simulink model "SOC_2023b"  (R2023b)
settings: Solver=VariableStepAuto  StartTime=0.0  StopTime=10.0  RelTol=1e-3
undefined symbols (not defined in the file or in its folder): Initial_SOC
(part 3 of 187)

== system SOC_2023b/SOC/Multi-Temperature SOC EKF1 ==
  note: McMaster Automotive Research Centre - MARC
      | Electrical Engineer: Romulo Navega Vieira, M.Sc and MBA - PhD Candidate
  Current : Inport
  Voltage : Inport  Port=2
  Temperature : Inport  Port=3
  Data Store Write1 : DataStoreWrite  DataStoreName=HVBat_SOCcell001  IndexOptions=Index vector (dialog)  Indices=1  OutputSizes=1
  Extended Kalman Filter - Multi Temperature EKF : Subsystem
  Gain : Gain  Gain=100
  Saturation : Saturate  UpperLimit=100  LowerLimit=0
  HVBat_SOCcell001 : Outport
  wires:
  Extended Kalman Filter - Multi Temperature EKF/1(SOC) -> Gain/1
  Voltage/1 -> Extended Kalman Filter - Multi Temperature EKF/2(Voltage)
  Current/1 -> Extended Kalman Filter - Multi Temperature EKF/1(Current)
  Temperature/1 -> Extended Kalman Filter - Multi Temperature EKF/3(Temperature)
  Gain/1 -> Saturation/1
  Saturation/1 -> HVBat_SOCcell001/1, Data Store Write1/1

== system SOC_2023b/SOC/Multi-Temperature SOC EKF10 ==
  note: McMaster Automotive Research Centre - MARC
      | Electrical Engineer: Romulo Navega Vieira, M.Sc and MBA - PhD Candidate
  Current : Inport
  Voltage : Inport  Port=2
  Temperature : Inport  Port=3
  Data Store Write1 : DataStoreWrite  DataStoreName=HVBat_SOCcell010  IndexOptions=Index vector (dialog)  Indices=1  OutputSizes=1
  Extended Kalman Filter - Multi Temperature EKF : Subsystem
  Gain : Gain  Gain=100
  Saturation : Saturate  UpperLimit=100  LowerLimit=0
  HVBat_SOCcell010 : Outport
  wires:
  Voltage/1 -> Extended Kalman Filter - Multi Temperature EKF/2(Voltage)
  Current/1 -> Extended Kalman Filter - Multi Temperature EKF/1(Current)
  Temperature/1 -> Extended Kalman Filter - Multi Temperature EKF/3(Temperature)
  Extended Kalman Filter - Multi Temperature EKF/1(SOC) -> Gain/1
  Gain/1 -> Saturation/1
  Saturation/1 -> HVBat_SOCcell010/1, Data Store Write1/1

== system SOC_2023b/SOC/Multi-Temperature SOC EKF100 ==
  note: McMaster Automotive Research Centre - MARC
      | Electrical Engineer: Romulo Navega Vieira, M.Sc and MBA - PhD Candidate
  Current : Inport
  Voltage : Inport  Port=2
  Temperature : Inport  Port=3
  Data Store Write1 : DataStoreWrite  DataStoreName=HVBat_SOCcell100  IndexOptions=Index vector (dialog)  Indices=1  OutputSizes=1
  Extended Kalman Filter - Multi Temperature EKF : Subsystem
  Gain : Gain  Gain=100
  Saturation : Saturate  UpperLimit=100  LowerLimit=0
  HVBat_SOCcell100 : Outport
  wires:
  Voltage/1 -> Extended Kalman Filter - Multi Temperature EKF/2(Voltage)
  Current/1 -> Extended Kalman Filter - Multi Temperature EKF/1(Current)
  Temperature/1 -> Extended Kalman Filter - Multi Temperature EKF/3(Temperature)
  Extended Kalman Filter - Multi Temperature EKF/1(SOC) -> Gain/1
  Gain/1 -> Saturation/1
  Saturation/1 -> HVBat_SOCcell100/1, Data Store Write1/1

== system SOC_2023b/SOC/Multi-Temperature SOC EKF101 ==
  note: McMaster Automotive Research Centre - MARC
      | Electrical Engineer: Romulo Navega Vieira, M.Sc and MBA - PhD Candidate
  Current : Inport
  Voltage : Inport  Port=2
  Temperature : Inport  Port=3
  Data Store Write1 : DataStoreWrite  DataStoreName=HVBat_SOCcell101  IndexOptions=Index vector (dialog)  Indices=1  OutputSizes=1
  Extended Kalman Filter - Multi Temperature EKF : Subsystem
  Gain : Gain  Gain=100
  Saturation : Saturate  UpperLimit=100  LowerLimit=0
  HVBat_SOCcell101 : Outport
  wires:
  Voltage/1 -> Extended Kalman Filter - Multi Temperature EKF/2(Voltage)
  Current/1 -> Extended Kalman Filter - Multi Temperature EKF/1(Current)
  Temperature/1 -> Extended Kalman Filter - Multi Temperature EKF/3(Temperature)
  Extended Kalman Filter - Multi Temperature EKF/1(SOC) -> Gain/1
  Gain/1 -> Saturation/1
  Saturation/1 -> HVBat_SOCcell101/1, Data Store Write1/1

== system SOC_2023b/SOC/Multi-Temperature SOC EKF102 ==
  note: McMaster Automotive Research Centre - MARC
      | Electrical Engineer: Romulo Navega Vieira, M.Sc and MBA - PhD Candidate
  Current : Inport
  Voltage : Inport  Port=2
  Temperature : Inport  Port=3
  Data Store Write1 : DataStoreWrite  DataStoreName=HVBat_SOCcell102  IndexOptions=Index vector (dialog)  Indices=1  OutputSizes=1
  Extended Kalman Filter - Multi Temperature EKF : Subsystem
  Gain : Gain  Gain=100
  Saturation : Saturate  UpperLimit=100  LowerLimit=0
  HVBat_SOCcell102 : Outport
  wires:
  Voltage/1 -> Extended Kalman Filter - Multi Temperature EKF/2(Voltage)
  Current/1 -> Extended Kalman Filter - Multi Temperature EKF/1(Current)
  Temperature/1 -> Extended Kalman Filter - Multi Temperature EKF/3(Temperature)
  Extended Kalman Filter - Multi Temperature EKF/1(SOC) -> Gain/1
  Gain/1 -> Saturation/1
  Saturation/1 -> HVBat_SOCcell102/1, Data Store Write1/1

== system SOC_2023b/SOC/Multi-Temperature SOC EKF103 ==
  note: McMaster Automotive Research Centre - MARC
      | Electrical Engineer: Romulo Navega Vieira, M.Sc and MBA - PhD Candidate
  Current : Inport
  Voltage : Inport  Port=2
  Temperature : Inport  Port=3
  Data Store Write1 : DataStoreWrite  DataStoreName=HVBat_SOCcell103  IndexOptions=Index vector (dialog)  Indices=1  OutputSizes=1
  Extended Kalman Filter - Multi Temperature EKF : Subsystem
  Gain : Gain  Gain=100
  Saturation : Saturate  UpperLimit=100  LowerLimit=0
  HVBat_SOCcell103 : Outport
  wires:
  Voltage/1 -> Extended Kalman Filter - Multi Temperature EKF/2(Voltage)
  Current/1 -> Extended Kalman Filter - Multi Temperature EKF/1(Current)
  Temperature/1 -> Extended Kalman Filter - Multi Temperature EKF/3(Temperature)
  Extended Kalman Filter - Multi Temperature EKF/1(SOC) -> Gain/1
  Gain/1 -> Saturation/1
  Saturation/1 -> HVBat_SOCcell103/1, Data Store Write1/1

== system SOC_2023b/SOC/Multi-Temperature SOC EKF104 ==
  note: McMaster Automotive Research Centre - MARC
      | Electrical Engineer: Romulo Navega Vieira, M.Sc and MBA - PhD Candidate
  Current : Inport
  Voltage : Inport  Port=2
  Temperature : Inport  Port=3
  Data Store Write1 : DataStoreWrite  DataStoreName=HVBat_SOCcell104  IndexOptions=Index vector (dialog)  Indices=1  OutputSizes=1
  Extended Kalman Filter - Multi Temperature EKF : Subsystem
  Gain : Gain  Gain=100
  Saturation : Saturate  UpperLimit=100  LowerLimit=0
  HVBat_SOCcell104 : Outport
  wires:
  Voltage/1 -> Extended Kalman Filter - Multi Temperature EKF/2(Voltage)
  Current/1 -> Extended Kalman Filter - Multi Temperature EKF/1(Current)
  Temperature/1 -> Extended Kalman Filter - Multi Temperature EKF/3(Temperature)
  Extended Kalman Filter - Multi Temperature EKF/1(SOC) -> Gain/1
  Gain/1 -> Saturation/1
  Saturation/1 -> HVBat_SOCcell104/1, Data Store Write1/1

== system SOC_2023b/SOC/Multi-Temperature SOC EKF105 ==
  note: McMaster Automotive Research Centre - MARC
      | Electrical Engineer: Romulo Navega Vieira, M.Sc and MBA - PhD Candidate
  Current : Inport
  Voltage : Inport  Port=2
  Temperature : Inport  Port=3
  Data Store Write1 : DataStoreWrite  DataStoreName=HVBat_SOCcell105  IndexOptions=Index vector (dialog)  Indices=1  OutputSizes=1
  Extended Kalman Filter - Multi Temperature EKF : Subsystem
  Gain : Gain  Gain=100
  Saturation : Saturate  UpperLimit=100  LowerLimit=0
  HVBat_SOCcell105 : Outport
  wires:
  Voltage/1 -> Extended Kalman Filter - Multi Temperature EKF/2(Voltage)
  Current/1 -> Extended Kalman Filter - Multi Temperature EKF/1(Current)
  Temperature/1 -> Extended Kalman Filter - Multi Temperature EKF/3(Temperature)
  Extended Kalman Filter - Multi Temperature EKF/1(SOC) -> Gain/1
  Gain/1 -> Saturation/1
  Saturation/1 -> HVBat_SOCcell105/1, Data Store Write1/1

== system SOC_2023b/SOC/Multi-Temperature SOC EKF11 ==
  note: McMaster Automotive Research Centre - MARC
      | Electrical Engineer: Romulo Navega Vieira, M.Sc and MBA - PhD Candidate
  Current : Inport
  Voltage : Inport  Port=2
  Temperature : Inport  Port=3
  Data Store Write1 : DataStoreWrite  DataStoreName=HVBat_SOCcell011  IndexOptions=Index vector (dialog)  Indices=1  OutputSizes=1
  Extended Kalman Filter - Multi Temperature EKF : Subsystem
  Gain : Gain  Gain=100
  Saturation : Saturate  UpperLimit=100  LowerLimit=0
  HVBat_SOCcell011 : Outport
  wires:
  Voltage/1 -> Extended Kalman Filter - Multi Temperature EKF/2(Voltage)
  Current/1 -> Extended Kalman Filter - Multi Temperature EKF/1(Current)
  Temperature/1 -> Extended Kalman Filter - Multi Temperature EKF/3(Temperature)
  Extended Kalman Filter - Multi Temperature EKF/1(SOC) -> Gain/1
  Gain/1 -> Saturation/1
  Saturation/1 -> HVBat_SOCcell011/1, Data Store Write1/1

== system SOC_2023b/SOC/Multi-Temperature SOC EKF12 ==
  note: McMaster Automotive Research Centre - MARC
      | Electrical Engineer: Romulo Navega Vieira, M.Sc and MBA - PhD Candidate
  Current : Inport
  Voltage : Inport  Port=2
  Temperature : Inport  Port=3
  Data Store Write1 : DataStoreWrite  DataStoreName=HVBat_SOCcell012  IndexOptions=Index vector (dialog)  Indices=1  OutputSizes=1
  Extended Kalman Filter - Multi Temperature EKF : Subsystem
  Gain : Gain  Gain=100
  Saturation : Saturate  UpperLimit=100  LowerLimit=0
  HVBat_SOCcell012 : Outport
  wires:
  Voltage/1 -> Extended Kalman Filter - Multi Temperature EKF/2(Voltage)
  Current/1 -> Extended Kalman Filter - Multi Temperature EKF/1(Current)
  Temperature/1 -> Extended Kalman Filter - Multi Temperature EKF/3(Temperature)
  Extended Kalman Filter - Multi Temperature EKF/1(SOC) -> Gain/1
  Gain/1 -> Saturation/1
  Saturation/1 -> HVBat_SOCcell012/1, Data Store Write1/1

== system SOC_2023b/SOC/Multi-Temperature SOC EKF13 ==
  note: McMaster Automotive Research Centre - MARC
      | Electrical Engineer: Romulo Navega Vieira, M.Sc and MBA - PhD Candidate
  Current : Inport
  Voltage : Inport  Port=2
  Temperature : Inport  Port=3
  Data Store Write1 : DataStoreWrite  DataStoreName=HVBat_SOCcell013  IndexOptions=Index vector (dialog)  Indices=1  OutputSizes=1
  Extended Kalman Filter - Multi Temperature EKF : Subsystem
  Gain : Gain  Gain=100
  Saturation : Saturate  UpperLimit=100  LowerLimit=0
  HVBat_SOCcell013 : Outport
  wires:
  Voltage/1 -> Extended Kalman Filter - Multi Temperature EKF/2(Voltage)
  Current/1 -> Extended Kalman Filter - Multi Temperature EKF/1(Current)
  Temperature/1 -> Extended Kalman Filter - Multi Temperature EKF/3(Temperature)
  Extended Kalman Filter - Multi Temperature EKF/1(SOC) -> Gain/1
  Gain/1 -> Saturation/1
  Saturation/1 -> HVBat_SOCcell013/1, Data Store Write1/1

== system SOC_2023b/SOC/Multi-Temperature SOC EKF14 ==
  note: McMaster Automotive Research Centre - MARC
      | Electrical Engineer: Romulo Navega Vieira, M.Sc and MBA - PhD Candidate
  Current : Inport
  Voltage : Inport  Port=2
  Temperature : Inport  Port=3
  Data Store Write1 : DataStoreWrite  DataStoreName=HVBat_SOCcell014  IndexOptions=Index vector (dialog)  Indices=1  OutputSizes=1
  Extended Kalman Filter - Multi Temperature EKF : Subsystem
  Gain : Gain  Gain=100
  Saturation : Saturate  UpperLimit=100  LowerLimit=0
  HVBat_SOCcell014 : Outport
  wires:
  Voltage/1 -> Extended Kalman Filter - Multi Temperature EKF/2(Voltage)
  Current/1 -> Extended Kalman Filter - Multi Temperature EKF/1(Current)
  Temperature/1 -> Extended Kalman Filter - Multi Temperature EKF/3(Temperature)
  Extended Kalman Filter - Multi Temperature EKF/1(SOC) -> Gain/1
  Gain/1 -> Saturation/1
  Saturation/1 -> HVBat_SOCcell014/1, Data Store Write1/1

== system SOC_2023b/SOC/Multi-Temperature SOC EKF15 ==
  note: McMaster Automotive Research Centre - MARC
      | Electrical Engineer: Romulo Navega Vieira, M.Sc and MBA - PhD Candidate
  Current : Inport
  Voltage : Inport  Port=2
  Temperature : Inport  Port=3
  Data Store Write1 : DataStoreWrite  DataStoreName=HVBat_SOCcell015  IndexOptions=Index vector (dialog)  Indices=1  OutputSizes=1
  Extended Kalman Filter - Multi Temperature EKF : Subsystem
  Gain : Gain  Gain=100
  Saturation : Saturate  UpperLimit=100  LowerLimit=0
  HVBat_SOCcell015 : Outport
  wires:
  Voltage/1 -> Extended Kalman Filter - Multi Temperature EKF/2(Voltage)
  Current/1 -> Extended Kalman Filter - Multi Temperature EKF/1(Current)
  Temperature/1 -> Extended Kalman Filter - Multi Temperature EKF/3(Temperature)
  Extended Kalman Filter - Multi Temperature EKF/1(SOC) -> Gain/1
  Gain/1 -> Saturation/1
  Saturation/1 -> HVBat_SOCcell015/1, Data Store Write1/1

== system SOC_2023b/SOC/Multi-Temperature SOC EKF16 ==
  note: McMaster Automotive Research Centre - MARC
      | Electrical Engineer: Romulo Navega Vieira, M.Sc and MBA - PhD Candidate
  Current : Inport
  Voltage : Inport  Port=2
  Temperature : Inport  Port=3
  Data Store Write1 : DataStoreWrite  DataStoreName=HVBat_SOCcell016  IndexOptions=Index vector (dialog)  Indices=1  OutputSizes=1
  Extended Kalman Filter - Multi Temperature EKF : Subsystem
  Gain : Gain  Gain=100
  Saturation : Saturate  UpperLimit=100  LowerLimit=0
  HVBat_SOCcell016 : Outport
  wires:
  Voltage/1 -> Extended Kalman Filter - Multi Temperature EKF/2(Voltage)
  Current/1 -> Extended Kalman Filter - Multi Temperature EKF/1(Current)
  Temperature/1 -> Extended Kalman Filter - Multi Temperature EKF/3(Temperature)
  Extended Kalman Filter - Multi Temperature EKF/1(SOC) -> Gain/1
  Gain/1 -> Saturation/1
  Saturation/1 -> HVBat_SOCcell016/1, Data Store Write1/1

== system SOC_2023b/SOC/Multi-Temperature SOC EKF17 ==
  note: McMaster Automotive Research Centre - MARC
      | Electrical Engineer: Romulo Navega Vieira, M.Sc and MBA - PhD Candidate
  Current : Inport
  Voltage : Inport  Port=2
  Temperature : Inport  Port=3
  Data Store Write1 : DataStoreWrite  DataStoreName=HVBat_SOCcell017  IndexOptions=Index vector (dialog)  Indices=1  OutputSizes=1
  Extended Kalman Filter - Multi Temperature EKF : Subsystem
  Gain : Gain  Gain=100
  Saturation : Saturate  UpperLimit=100  LowerLimit=0
  HVBat_SOCcell017 : Outport
  wires:
  Voltage/1 -> Extended Kalman Filter - Multi Temperature EKF/2(Voltage)
  Current/1 -> Extended Kalman Filter - Multi Temperature EKF/1(Current)
  Temperature/1 -> Extended Kalman Filter - Multi Temperature EKF/3(Temperature)
  Extended Kalman Filter - Multi Temperature EKF/1(SOC) -> Gain/1
  Gain/1 -> Saturation/1
  Saturation/1 -> HVBat_SOCcell017/1, Data Store Write1/1

== system SOC_2023b/SOC/Multi-Temperature SOC EKF18 ==
  note: McMaster Automotive Research Centre - MARC
      | Electrical Engineer: Romulo Navega Vieira, M.Sc and MBA - PhD Candidate
  Current : Inport
  Voltage : Inport  Port=2
  Temperature : Inport  Port=3
  Data Store Write1 : DataStoreWrite  DataStoreName=HVBat_SOCcell018  IndexOptions=Index vector (dialog)  Indices=1  OutputSizes=1
  Extended Kalman Filter - Multi Temperature EKF : Subsystem
  Gain : Gain  Gain=100
  Saturation : Saturate  UpperLimit=100  LowerLimit=0
  HVBat_SOCcell018 : Outport
  wires:
  Voltage/1 -> Extended Kalman Filter - Multi Temperature EKF/2(Voltage)
  Current/1 -> Extended Kalman Filter - Multi Temperature EKF/1(Current)
  Temperature/1 -> Extended Kalman Filter - Multi Temperature EKF/3(Temperature)
  Extended Kalman Filter - Multi Temperature EKF/1(SOC) -> Gain/1
  Gain/1 -> Saturation/1
  Saturation/1 -> HVBat_SOCcell018/1, Data Store Write1/1

== system SOC_2023b/SOC/Multi-Temperature SOC EKF19 ==
  note: McMaster Automotive Research Centre - MARC
      | Electrical Engineer: Romulo Navega Vieira, M.Sc and MBA - PhD Candidate
  Current : Inport
  Voltage : Inport  Port=2
  Temperature : Inport  Port=3
  Data Store Write1 : DataStoreWrite  DataStoreName=HVBat_SOCcell019  IndexOptions=Index vector (dialog)  Indices=1  OutputSizes=1
  Extended Kalman Filter - Multi Temperature EKF : Subsystem
  Gain : Gain  Gain=100
  Saturation : Saturate  UpperLimit=100  LowerLimit=0
  HVBat_SOCcell019 : Outport
  wires:
  Voltage/1 -> Extended Kalman Filter - Multi Temperature EKF/2(Voltage)
  Current/1 -> Extended Kalman Filter - Multi Temperature EKF/1(Current)
  Temperature/1 -> Extended Kalman Filter - Multi Temperature EKF/3(Temperature)
  Extended Kalman Filter - Multi Temperature EKF/1(SOC) -> Gain/1
  Gain/1 -> Saturation/1
  Saturation/1 -> HVBat_SOCcell019/1, Data Store Write1/1

== system SOC_2023b/SOC/Multi-Temperature SOC EKF2 ==
  note: McMaster Automotive Research Centre - MARC
      | Electrical Engineer: Romulo Navega Vieira, M.Sc and MBA - PhD Candidate
  Current : Inport
  Voltage : Inport  Port=2
  Temperature : Inport  Port=3
  Data Store Write1 : DataStoreWrite  DataStoreName=HVBat_SOCcell002  IndexOptions=Index vector (dialog)  Indices=1  OutputSizes=1
  Extended Kalman Filter - Multi Temperature EKF : Subsystem
  Gain : Gain  Gain=100
  Saturation : Saturate  UpperLimit=100  LowerLimit=0
  HVBat_SOCcell002 : Outport
  wires:
  Voltage/1 -> Extended Kalman Filter - Multi Temperature EKF/2(Voltage)
  Current/1 -> Extended Kalman Filter - Multi Temperature EKF/1(Current)
  Temperature/1 -> Extended Kalman Filter - Multi Temperature EKF/3(Temperature)
  Extended Kalman Filter - Multi Temperature EKF/1(SOC) -> Gain/1
  Gain/1 -> Saturation/1
  Saturation/1 -> HVBat_SOCcell002/1, Data Store Write1/1

== system SOC_2023b/SOC/Multi-Temperature SOC EKF20 ==
  note: McMaster Automotive Research Centre - MARC
      | Electrical Engineer: Romulo Navega Vieira, M.Sc and MBA - PhD Candidate
  Current : Inport
  Voltage : Inport  Port=2
  Temperature : Inport  Port=3
  Data Store Write1 : DataStoreWrite  DataStoreName=HVBat_SOCcell020  IndexOptions=Index vector (dialog)  Indices=1  OutputSizes=1
  Extended Kalman Filter - Multi Temperature EKF : Subsystem
  Gain : Gain  Gain=100
  Saturation : Saturate  UpperLimit=100  LowerLimit=0
  HVBat_SOCcell020 : Outport
  wires:
  Voltage/1 -> Extended Kalman Filter - Multi Temperature EKF/2(Voltage)
  Current/1 -> Extended Kalman Filter - Multi Temperature EKF/1(Current)
  Temperature/1 -> Extended Kalman Filter - Multi Temperature EKF/3(Temperature)
  Extended Kalman Filter - Multi Temperature EKF/1(SOC) -> Gain/1
  Gain/1 -> Saturation/1
  Saturation/1 -> HVBat_SOCcell020/1, Data Store Write1/1

== system SOC_2023b/SOC/Multi-Temperature SOC EKF21 ==
  note: McMaster Automotive Research Centre - MARC
      | Electrical Engineer: Romulo Navega Vieira, M.Sc and MBA - PhD Candidate
  Current : Inport
  Voltage : Inport  Port=2
  Temperature : Inport  Port=3
  Data Store Write1 : DataStoreWrite  DataStoreName=HVBat_SOCcell021  IndexOptions=Index vector (dialog)  Indices=1  OutputSizes=1
  Extended Kalman Filter - Multi Temperature EKF : Subsystem
  Gain : Gain  Gain=100
  Saturation : Saturate  UpperLimit=100  LowerLimit=0
  HVBat_SOCcell021 : Outport
  wires:
  Extended Kalman Filter - Multi Temperature EKF/1(SOC) -> Gain/1
  Voltage/1 -> Extended Kalman Filter - Multi Temperature EKF/2(Voltage)
  Current/1 -> Extended Kalman Filter - Multi Temperature EKF/1(Current)
  Temperature/1 -> Extended Kalman Filter - Multi Temperature EKF/3(Temperature)
  Gain/1 -> Saturation/1
  Saturation/1 -> HVBat_SOCcell021/1, Data Store Write1/1

== system SOC_2023b/SOC/Multi-Temperature SOC EKF22 ==
  note: McMaster Automotive Research Centre - MARC
      | Electrical Engineer: Romulo Navega Vieira, M.Sc and MBA - PhD Candidate
  Current : Inport
  Voltage : Inport  Port=2
  Temperature : Inport  Port=3
  Data Store Write1 : DataStoreWrite  DataStoreName=HVBat_SOCcell022  IndexOptions=Index vector (dialog)  Indices=1  OutputSizes=1
  Extended Kalman Filter - Multi Temperature EKF : Subsystem
  Gain : Gain  Gain=100
  Saturation : Saturate  UpperLimit=100  LowerLimit=0
  HVBat_SOCcell022 : Outport
  wires:
  Voltage/1 -> Extended Kalman Filter - Multi Temperature EKF/2(Voltage)
  Current/1 -> Extended Kalman Filter - Multi Temperature EKF/1(Current)
  Temperature/1 -> Extended Kalman Filter - Multi Temperature EKF/3(Temperature)
  Extended Kalman Filter - Multi Temperature EKF/1(SOC) -> Gain/1
  Gain/1 -> Saturation/1
  Saturation/1 -> HVBat_SOCcell022/1, Data Store Write1/1

== system SOC_2023b/SOC/Multi-Temperature SOC EKF23 ==
  note: McMaster Automotive Research Centre - MARC
      | Electrical Engineer: Romulo Navega Vieira, M.Sc and MBA - PhD Candidate
  Current : Inport
  Voltage : Inport  Port=2
  Temperature : Inport  Port=3
  Data Store Write1 : DataStoreWrite  DataStoreName=HVBat_SOCcell023  IndexOptions=Index vector (dialog)  Indices=1  OutputSizes=1
  Extended Kalman Filter - Multi Temperature EKF : Subsystem
  Gain : Gain  Gain=100
  Saturation : Saturate  UpperLimit=100  LowerLimit=0
  HVBat_SOCcell023 : Outport
  wires:
  Voltage/1 -> Extended Kalman Filter - Multi Temperature EKF/2(Voltage)
  Current/1 -> Extended Kalman Filter - Multi Temperature EKF/1(Current)
  Temperature/1 -> Extended Kalman Filter - Multi Temperature EKF/3(Temperature)
  Extended Kalman Filter - Multi Temperature EKF/1(SOC) -> Gain/1
  Gain/1 -> Saturation/1
  Saturation/1 -> HVBat_SOCcell023/1, Data Store Write1/1

== system SOC_2023b/SOC/Multi-Temperature SOC EKF24 ==
  note: McMaster Automotive Research Centre - MARC
      | Electrical Engineer: Romulo Navega Vieira, M.Sc and MBA - PhD Candidate
  Current : Inport
  Voltage : Inport  Port=2
  Temperature : Inport  Port=3
  Data Store Write1 : DataStoreWrite  DataStoreName=HVBat_SOCcell024  IndexOptions=Index vector (dialog)  Indices=1  OutputSizes=1
  Extended Kalman Filter - Multi Temperature EKF : Subsystem
  Gain : Gain  Gain=100
  Saturation : Saturate  UpperLimit=100  LowerLimit=0
  HVBat_SOCcell024 : Outport
  wires:
  Voltage/1 -> Extended Kalman Filter - Multi Temperature EKF/2(Voltage)
  Current/1 -> Extended Kalman Filter - Multi Temperature EKF/1(Current)
  Temperature/1 -> Extended Kalman Filter - Multi Temperature EKF/3(Temperature)
  Extended Kalman Filter - Multi Temperature EKF/1(SOC) -> Gain/1
  Gain/1 -> Saturation/1
  Saturation/1 -> HVBat_SOCcell024/1, Data Store Write1/1

== system SOC_2023b/SOC/Multi-Temperature SOC EKF25 ==
  note: McMaster Automotive Research Centre - MARC
      | Electrical Engineer: Romulo Navega Vieira, M.Sc and MBA - PhD Candidate
  Current : Inport
  Voltage : Inport  Port=2
  Temperature : Inport  Port=3
  Data Store Write1 : DataStoreWrite  DataStoreName=HVBat_SOCcell025  IndexOptions=Index vector (dialog)  Indices=1  OutputSizes=1
  Extended Kalman Filter - Multi Temperature EKF : Subsystem
  Gain : Gain  Gain=100
  Saturation : Saturate  UpperLimit=100  LowerLimit=0
  HVBat_SOCcell025 : Outport
  wires:
  Voltage/1 -> Extended Kalman Filter - Multi Temperature EKF/2(Voltage)
  Current/1 -> Extended Kalman Filter - Multi Temperature EKF/1(Current)
  Temperature/1 -> Extended Kalman Filter - Multi Temperature EKF/3(Temperature)
  Extended Kalman Filter - Multi Temperature EKF/1(SOC) -> Gain/1
  Gain/1 -> Saturation/1
  Saturation/1 -> HVBat_SOCcell025/1, Data Store Write1/1

== system SOC_2023b/SOC/Multi-Temperature SOC EKF26 ==
  note: McMaster Automotive Research Centre - MARC
      | Electrical Engineer: Romulo Navega Vieira, M.Sc and MBA - PhD Candidate
  Current : Inport
  Voltage : Inport  Port=2
  Temperature : Inport  Port=3
  Data Store Write1 : DataStoreWrite  DataStoreName=HVBat_SOCcell026  IndexOptions=Index vector (dialog)  Indices=1  OutputSizes=1
  Extended Kalman Filter - Multi Temperature EKF : Subsystem
  Gain : Gain  Gain=100
  Saturation : Saturate  UpperLimit=100  LowerLimit=0
  HVBat_SOCcell026 : Outport
  wires:
  Voltage/1 -> Extended Kalman Filter - Multi Temperature EKF/2(Voltage)
  Current/1 -> Extended Kalman Filter - Multi Temperature EKF/1(Current)
  Temperature/1 -> Extended Kalman Filter - Multi Temperature EKF/3(Temperature)
  Extended Kalman Filter - Multi Temperature EKF/1(SOC) -> Gain/1
  Gain/1 -> Saturation/1
  Saturation/1 -> HVBat_SOCcell026/1, Data Store Write1/1

== system SOC_2023b/SOC/Multi-Temperature SOC EKF27 ==
  note: McMaster Automotive Research Centre - MARC
      | Electrical Engineer: Romulo Navega Vieira, M.Sc and MBA - PhD Candidate
  Current : Inport
  Voltage : Inport  Port=2
  Temperature : Inport  Port=3
  Data Store Write1 : DataStoreWrite  DataStoreName=HVBat_SOCcell027  IndexOptions=Index vector (dialog)  Indices=1  OutputSizes=1
  Extended Kalman Filter - Multi Temperature EKF : Subsystem
  Gain : Gain  Gain=100
  Saturation : Saturate  UpperLimit=100  LowerLimit=0
  HVBat_SOCcell027 : Outport
  wires:
  Voltage/1 -> Extended Kalman Filter - Multi Temperature EKF/2(Voltage)
  Current/1 -> Extended Kalman Filter - Multi Temperature EKF/1(Current)
  Temperature/1 -> Extended Kalman Filter - Multi Temperature EKF/3(Temperature)
  Extended Kalman Filter - Multi Temperature EKF/1(SOC) -> Gain/1
  Gain/1 -> Saturation/1
  Saturation/1 -> HVBat_SOCcell027/1, Data Store Write1/1

== system SOC_2023b/SOC/Multi-Temperature SOC EKF28 ==
  note: McMaster Automotive Research Centre - MARC
      | Electrical Engineer: Romulo Navega Vieira, M.Sc and MBA - PhD Candidate
  Current : Inport
  Voltage : Inport  Port=2
  Temperature : Inport  Port=3
  Data Store Write1 : DataStoreWrite  DataStoreName=HVBat_SOCcell028  IndexOptions=Index vector (dialog)  Indices=1  OutputSizes=1
  Extended Kalman Filter - Multi Temperature EKF : Subsystem
  Gain : Gain  Gain=100
  Saturation : Saturate  UpperLimit=100  LowerLimit=0
  HVBat_SOCcell028 : Outport
  wires:
  Voltage/1 -> Extended Kalman Filter - Multi Temperature EKF/2(Voltage)
  Current/1 -> Extended Kalman Filter - Multi Temperature EKF/1(Current)
  Temperature/1 -> Extended Kalman Filter - Multi Temperature EKF/3(Temperature)
  Extended Kalman Filter - Multi Temperature EKF/1(SOC) -> Gain/1
  Gain/1 -> Saturation/1
  Saturation/1 -> HVBat_SOCcell028/1, Data Store Write1/1

== system SOC_2023b/SOC/Multi-Temperature SOC EKF29 ==
  note: McMaster Automotive Research Centre - MARC
      | Electrical Engineer: Romulo Navega Vieira, M.Sc and MBA - PhD Candidate
  Current : Inport
  Voltage : Inport  Port=2
  Temperature : Inport  Port=3
  Data Store Write1 : DataStoreWrite  DataStoreName=HVBat_SOCcell029  IndexOptions=Index vector (dialog)  Indices=1  OutputSizes=1
  Extended Kalman Filter - Multi Temperature EKF : Subsystem
  Gain : Gain  Gain=100
  Saturation : Saturate  UpperLimit=100  LowerLimit=0
  HVBat_SOCcell029 : Outport
  wires:
  Voltage/1 -> Extended Kalman Filter - Multi Temperature EKF/2(Voltage)
  Current/1 -> Extended Kalman Filter - Multi Temperature EKF/1(Current)
  Temperature/1 -> Extended Kalman Filter - Multi Temperature EKF/3(Temperature)
  Extended Kalman Filter - Multi Temperature EKF/1(SOC) -> Gain/1
  Gain/1 -> Saturation/1
  Saturation/1 -> HVBat_SOCcell029/1, Data Store Write1/1

== system SOC_2023b/SOC/Multi-Temperature SOC EKF3 ==
  note: McMaster Automotive Research Centre - MARC
      | Electrical Engineer: Romulo Navega Vieira, M.Sc and MBA - PhD Candidate
  Current : Inport
  Voltage : Inport  Port=2
  Temperature : Inport  Port=3
  Data Store Write1 : DataStoreWrite  DataStoreName=HVBat_SOCcell003  IndexOptions=Index vector (dialog)  Indices=1  OutputSizes=1
  Extended Kalman Filter - Multi Temperature EKF : Subsystem
  Gain : Gain  Gain=100
  Saturation : Saturate  UpperLimit=100  LowerLimit=0
  HVBat_SOCcell003 : Outport
  wires:
  Voltage/1 -> Extended Kalman Filter - Multi Temperature EKF/2(Voltage)
  Current/1 -> Extended Kalman Filter - Multi Temperature EKF/1(Current)
  Temperature/1 -> Extended Kalman Filter - Multi Temperature EKF/3(Temperature)
  Extended Kalman Filter - Multi Temperature EKF/1(SOC) -> Gain/1
  Gain/1 -> Saturation/1
  Saturation/1 -> HVBat_SOCcell003/1, Data Store Write1/1

== system SOC_2023b/SOC/Multi-Temperature SOC EKF30 ==
  note: McMaster Automotive Research Centre - MARC
      | Electrical Engineer: Romulo Navega Vieira, M.Sc and MBA - PhD Candidate
  Current : Inport
  Voltage : Inport  Port=2
  Temperature : Inport  Port=3
  Data Store Write1 : DataStoreWrite  DataStoreName=HVBat_SOCcell030  IndexOptions=Index vector (dialog)  Indices=1  OutputSizes=1
  Extended Kalman Filter - Multi Temperature EKF : Subsystem
  Gain : Gain  Gain=100
  Saturation : Saturate  UpperLimit=100  LowerLimit=0
  HVBat_SOCcell030 : Outport
  wires:
  Voltage/1 -> Extended Kalman Filter - Multi Temperature EKF/2(Voltage)
  Current/1 -> Extended Kalman Filter - Multi Temperature EKF/1(Current)
  Temperature/1 -> Extended Kalman Filter - Multi Temperature EKF/3(Temperature)
  Extended Kalman Filter - Multi Temperature EKF/1(SOC) -> Gain/1
  Gain/1 -> Saturation/1
  Saturation/1 -> HVBat_SOCcell030/1, Data Store Write1/1

== system SOC_2023b/SOC/Multi-Temperature SOC EKF31 ==
  note: McMaster Automotive Research Centre - MARC
      | Electrical Engineer: Romulo Navega Vieira, M.Sc and MBA - PhD Candidate
  Current : Inport
  Voltage : Inport  Port=2
  Temperature : Inport  Port=3
  Data Store Write1 : DataStoreWrite  DataStoreName=HVBat_SOCcell031  IndexOptions=Index vector (dialog)  Indices=1  OutputSizes=1
  Extended Kalman Filter - Multi Temperature EKF : Subsystem
  Gain : Gain  Gain=100
  Saturation : Saturate  UpperLimit=100  LowerLimit=0
  HVBat_SOCcell031 : Outport
  wires:
  Voltage/1 -> Extended Kalman Filter - Multi Temperature EKF/2(Voltage)
  Current/1 -> Extended Kalman Filter - Multi Temperature EKF/1(Current)
  Temperature/1 -> Extended Kalman Filter - Multi Temperature EKF/3(Temperature)
  Extended Kalman Filter - Multi Temperature EKF/1(SOC) -> Gain/1
  Gain/1 -> Saturation/1
  Saturation/1 -> HVBat_SOCcell031/1, Data Store Write1/1

== system SOC_2023b/SOC/Multi-Temperature SOC EKF32 ==
  note: McMaster Automotive Research Centre - MARC
      | Electrical Engineer: Romulo Navega Vieira, M.Sc and MBA - PhD Candidate
  Current : Inport
  Voltage : Inport  Port=2
  Temperature : Inport  Port=3
  Data Store Write1 : DataStoreWrite  DataStoreName=HVBat_SOCcell032  IndexOptions=Index vector (dialog)  Indices=1  OutputSizes=1
  Extended Kalman Filter - Multi Temperature EKF : Subsystem
  Gain : Gain  Gain=100
  Saturation : Saturate  UpperLimit=100  LowerLimit=0
  HVBat_SOCcell032 : Outport
  wires:
  Voltage/1 -> Extended Kalman Filter - Multi Temperature EKF/2(Voltage)
  Current/1 -> Extended Kalman Filter - Multi Temperature EKF/1(Current)
  Temperature/1 -> Extended Kalman Filter - Multi Temperature EKF/3(Temperature)
  Extended Kalman Filter - Multi Temperature EKF/1(SOC) -> Gain/1
  Gain/1 -> Saturation/1
  Saturation/1 -> HVBat_SOCcell032/1, Data Store Write1/1

== system SOC_2023b/SOC/Multi-Temperature SOC EKF33 ==
  note: McMaster Automotive Research Centre - MARC
      | Electrical Engineer: Romulo Navega Vieira, M.Sc and MBA - PhD Candidate
  Current : Inport
  Voltage : Inport  Port=2
  Temperature : Inport  Port=3
  Data Store Write1 : DataStoreWrite  DataStoreName=HVBat_SOCcell033  IndexOptions=Index vector (dialog)  Indices=1  OutputSizes=1
  Extended Kalman Filter - Multi Temperature EKF : Subsystem
  Gain : Gain  Gain=100
  Saturation : Saturate  UpperLimit=100  LowerLimit=0
  HVBat_SOCcell033 : Outport
  wires:
  Voltage/1 -> Extended Kalman Filter - Multi Temperature EKF/2(Voltage)
  Current/1 -> Extended Kalman Filter - Multi Temperature EKF/1(Current)
  Temperature/1 -> Extended Kalman Filter - Multi Temperature EKF/3(Temperature)
  Extended Kalman Filter - Multi Temperature EKF/1(SOC) -> Gain/1
  Gain/1 -> Saturation/1
  Saturation/1 -> HVBat_SOCcell033/1, Data Store Write1/1

== system SOC_2023b/SOC/Multi-Temperature SOC EKF34 ==
  note: McMaster Automotive Research Centre - MARC
      | Electrical Engineer: Romulo Navega Vieira, M.Sc and MBA - PhD Candidate
  Current : Inport
  Voltage : Inport  Port=2
  Temperature : Inport  Port=3
  Data Store Write1 : DataStoreWrite  DataStoreName=HVBat_SOCcell034  IndexOptions=Index vector (dialog)  Indices=1  OutputSizes=1
  Extended Kalman Filter - Multi Temperature EKF : Subsystem
  Gain : Gain  Gain=100
  Saturation : Saturate  UpperLimit=100  LowerLimit=0
  HVBat_SOCcell034 : Outport
  wires:
  Voltage/1 -> Extended Kalman Filter - Multi Temperature EKF/2(Voltage)
  Current/1 -> Extended Kalman Filter - Multi Temperature EKF/1(Current)
  Temperature/1 -> Extended Kalman Filter - Multi Temperature EKF/3(Temperature)
  Extended Kalman Filter - Multi Temperature EKF/1(SOC) -> Gain/1
  Gain/1 -> Saturation/1
  Saturation/1 -> HVBat_SOCcell034/1, Data Store Write1/1

== system SOC_2023b/SOC/Multi-Temperature SOC EKF35 ==
  note: McMaster Automotive Research Centre - MARC
      | Electrical Engineer: Romulo Navega Vieira, M.Sc and MBA - PhD Candidate
  Current : Inport
  Voltage : Inport  Port=2
  Temperature : Inport  Port=3
  Data Store Write1 : DataStoreWrite  DataStoreName=HVBat_SOCcell035  IndexOptions=Index vector (dialog)  Indices=1  OutputSizes=1
  Extended Kalman Filter - Multi Temperature EKF : Subsystem
  Gain : Gain  Gain=100
  Saturation : Saturate  UpperLimit=100  LowerLimit=0
  HVBat_SOCcell035 : Outport
  wires:
  Voltage/1 -> Extended Kalman Filter - Multi Temperature EKF/2(Voltage)
  Current/1 -> Extended Kalman Filter - Multi Temperature EKF/1(Current)
  Temperature/1 -> Extended Kalman Filter - Multi Temperature EKF/3(Temperature)
  Extended Kalman Filter - Multi Temperature EKF/1(SOC) -> Gain/1
  Gain/1 -> Saturation/1
  Saturation/1 -> HVBat_SOCcell035/1, Data Store Write1/1

== system SOC_2023b/SOC/Multi-Temperature SOC EKF36 ==
  note: McMaster Automotive Research Centre - MARC
      | Electrical Engineer: Romulo Navega Vieira, M.Sc and MBA - PhD Candidate
  Current : Inport
  Voltage : Inport  Port=2
  Temperature : Inport  Port=3
  Data Store Write1 : DataStoreWrite  DataStoreName=HVBat_SOCcell036  IndexOptions=Index vector (dialog)  Indices=1  OutputSizes=1
  Extended Kalman Filter - Multi Temperature EKF : Subsystem
  Gain : Gain  Gain=100
  Saturation : Saturate  UpperLimit=100  LowerLimit=0
  HVBat_SOCcell036 : Outport
  wires:
  Voltage/1 -> Extended Kalman Filter - Multi Temperature EKF/2(Voltage)
  Current/1 -> Extended Kalman Filter - Multi Temperature EKF/1(Current)
  Temperature/1 -> Extended Kalman Filter - Multi Temperature EKF/3(Temperature)
  Extended Kalman Filter - Multi Temperature EKF/1(SOC) -> Gain/1
  Gain/1 -> Saturation/1
  Saturation/1 -> HVBat_SOCcell036/1, Data Store Write1/1

== system SOC_2023b/SOC/Multi-Temperature SOC EKF37 ==
  note: McMaster Automotive Research Centre - MARC
      | Electrical Engineer: Romulo Navega Vieira, M.Sc and MBA - PhD Candidate
  Current : Inport
  Voltage : Inport  Port=2
  Temperature : Inport  Port=3
  Data Store Write1 : DataStoreWrite  DataStoreName=HVBat_SOCcell037  IndexOptions=Index vector (dialog)  Indices=1  OutputSizes=1
  Extended Kalman Filter - Multi Temperature EKF : Subsystem
  Gain : Gain  Gain=100
  Saturation : Saturate  UpperLimit=100  LowerLimit=0
  HVBat_SOCcell037 : Outport
  wires:
  Voltage/1 -> Extended Kalman Filter - Multi Temperature EKF/2(Voltage)
  Current/1 -> Extended Kalman Filter - Multi Temperature EKF/1(Current)
  Temperature/1 -> Extended Kalman Filter - Multi Temperature EKF/3(Temperature)
  Extended Kalman Filter - Multi Temperature EKF/1(SOC) -> Gain/1
  Gain/1 -> Saturation/1
  Saturation/1 -> HVBat_SOCcell037/1, Data Store Write1/1

== system SOC_2023b/SOC/Multi-Temperature SOC EKF38 ==
  note: McMaster Automotive Research Centre - MARC
      | Electrical Engineer: Romulo Navega Vieira, M.Sc and MBA - PhD Candidate
  Current : Inport
  Voltage : Inport  Port=2
  Temperature : Inport  Port=3
  Data Store Write1 : DataStoreWrite  DataStoreName=HVBat_SOCcell038  IndexOptions=Index vector (dialog)  Indices=1  OutputSizes=1
  Extended Kalman Filter - Multi Temperature EKF : Subsystem
  Gain : Gain  Gain=100
  Saturation : Saturate  UpperLimit=100  LowerLimit=0
  HVBat_SOCcell038 : Outport
  wires:
  Voltage/1 -> Extended Kalman Filter - Multi Temperature EKF/2(Voltage)
  Current/1 -> Extended Kalman Filter - Multi Temperature EKF/1(Current)
  Temperature/1 -> Extended Kalman Filter - Multi Temperature EKF/3(Temperature)
  Extended Kalman Filter - Multi Temperature EKF/1(SOC) -> Gain/1
  Gain/1 -> Saturation/1
  Saturation/1 -> HVBat_SOCcell038/1, Data Store Write1/1

== system SOC_2023b/SOC/Multi-Temperature SOC EKF39 ==
  note: McMaster Automotive Research Centre - MARC
      | Electrical Engineer: Romulo Navega Vieira, M.Sc and MBA - PhD Candidate
  Current : Inport
  Voltage : Inport  Port=2
  Temperature : Inport  Port=3
  Data Store Write1 : DataStoreWrite  DataStoreName=HVBat_SOCcell039  IndexOptions=Index vector (dialog)  Indices=1  OutputSizes=1
  Extended Kalman Filter - Multi Temperature EKF : Subsystem
  Gain : Gain  Gain=100
  Saturation : Saturate  UpperLimit=100  LowerLimit=0
  HVBat_SOCcell039 : Outport
  wires:
  Voltage/1 -> Extended Kalman Filter - Multi Temperature EKF/2(Voltage)
  Current/1 -> Extended Kalman Filter - Multi Temperature EKF/1(Current)
  Temperature/1 -> Extended Kalman Filter - Multi Temperature EKF/3(Temperature)
  Extended Kalman Filter - Multi Temperature EKF/1(SOC) -> Gain/1
  Gain/1 -> Saturation/1
  Saturation/1 -> HVBat_SOCcell039/1, Data Store Write1/1

== system SOC_2023b/SOC/Multi-Temperature SOC EKF4 ==
  note: McMaster Automotive Research Centre - MARC
      | Electrical Engineer: Romulo Navega Vieira, M.Sc and MBA - PhD Candidate
  Current : Inport
  Voltage : Inport  Port=2
  Temperature : Inport  Port=3
  Data Store Write1 : DataStoreWrite  DataStoreName=HVBat_SOCcell004  IndexOptions=Index vector (dialog)  Indices=1  OutputSizes=1
  Extended Kalman Filter - Multi Temperature EKF : Subsystem
  Gain : Gain  Gain=100
  Saturation : Saturate  UpperLimit=100  LowerLimit=0
  HVBat_SOCcell004 : Outport
  wires:
  Voltage/1 -> Extended Kalman Filter - Multi Temperature EKF/2(Voltage)
  Current/1 -> Extended Kalman Filter - Multi Temperature EKF/1(Current)
  Temperature/1 -> Extended Kalman Filter - Multi Temperature EKF/3(Temperature)
  Extended Kalman Filter - Multi Temperature EKF/1(SOC) -> Gain/1
  Gain/1 -> Saturation/1
  Saturation/1 -> HVBat_SOCcell004/1, Data Store Write1/1

== system SOC_2023b/SOC/Multi-Temperature SOC EKF40 ==
  note: McMaster Automotive Research Centre - MARC
      | Electrical Engineer: Romulo Navega Vieira, M.Sc and MBA - PhD Candidate
  Current : Inport
  Voltage : Inport  Port=2
  Temperature : Inport  Port=3
  Data Store Write1 : DataStoreWrite  DataStoreName=HVBat_SOCcell040  IndexOptions=Index vector (dialog)  Indices=1  OutputSizes=1
  Extended Kalman Filter - Multi Temperature EKF : Subsystem
  Gain : Gain  Gain=100
  Saturation : Saturate  UpperLimit=100  LowerLimit=0
  HVBat_SOCcell040 : Outport
  wires:
  Extended Kalman Filter - Multi Temperature EKF/1(SOC) -> Gain/1
  Voltage/1 -> Extended Kalman Filter - Multi Temperature EKF/2(Voltage)
  Current/1 -> Extended Kalman Filter - Multi Temperature EKF/1(Current)
  Temperature/1 -> Extended Kalman Filter - Multi Temperature EKF/3(Temperature)
  Gain/1 -> Saturation/1
  Saturation/1 -> HVBat_SOCcell040/1, Data Store Write1/1

== system SOC_2023b/SOC/Multi-Temperature SOC EKF41 ==
  note: McMaster Automotive Research Centre - MARC
      | Electrical Engineer: Romulo Navega Vieira, M.Sc and MBA - PhD Candidate
  Current : Inport
  Voltage : Inport  Port=2
  Temperature : Inport  Port=3
  Data Store Write1 : DataStoreWrite  DataStoreName=HVBat_SOCcell041  IndexOptions=Index vector (dialog)  Indices=1  OutputSizes=1
  Extended Kalman Filter - Multi Temperature EKF : Subsystem
  Gain : Gain  Gain=100
  Saturation : Saturate  UpperLimit=100  LowerLimit=0
  HVBat_SOCcell041 : Outport
  wires:
  Voltage/1 -> Extended Kalman Filter - Multi Temperature EKF/2(Voltage)
  Current/1 -> Extended Kalman Filter - Multi Temperature EKF/1(Current)
  Temperature/1 -> Extended Kalman Filter - Multi Temperature EKF/3(Temperature)
  Extended Kalman Filter - Multi Temperature EKF/1(SOC) -> Gain/1
  Gain/1 -> Saturation/1
  Saturation/1 -> HVBat_SOCcell041/1, Data Store Write1/1

== system SOC_2023b/SOC/Multi-Temperature SOC EKF42 ==
  note: McMaster Automotive Research Centre - MARC
      | Electrical Engineer: Romulo Navega Vieira, M.Sc and MBA - PhD Candidate
  Current : Inport
  Voltage : Inport  Port=2
  Temperature : Inport  Port=3
  Data Store Write1 : DataStoreWrite  DataStoreName=HVBat_SOCcell042  IndexOptions=Index vector (dialog)  Indices=1  OutputSizes=1
  Extended Kalman Filter - Multi Temperature EKF : Subsystem
  Gain : Gain  Gain=100
  Saturation : Saturate  UpperLimit=100  LowerLimit=0
  HVBat_SOCcell042 : Outport
  wires:
  Voltage/1 -> Extended Kalman Filter - Multi Temperature EKF/2(Voltage)
  Current/1 -> Extended Kalman Filter - Multi Temperature EKF/1(Current)
  Temperature/1 -> Extended Kalman Filter - Multi Temperature EKF/3(Temperature)
  Extended Kalman Filter - Multi Temperature EKF/1(SOC) -> Gain/1
  Gain/1 -> Saturation/1
  Saturation/1 -> HVBat_SOCcell042/1, Data Store Write1/1

== system SOC_2023b/SOC/Multi-Temperature SOC EKF43 ==
  note: McMaster Automotive Research Centre - MARC
      | Electrical Engineer: Romulo Navega Vieira, M.Sc and MBA - PhD Candidate
  Current : Inport
  Voltage : Inport  Port=2
  Temperature : Inport  Port=3
  Data Store Write1 : DataStoreWrite  DataStoreName=HVBat_SOCcell043  IndexOptions=Index vector (dialog)  Indices=1  OutputSizes=1
  Extended Kalman Filter - Multi Temperature EKF : Subsystem
  Gain : Gain  Gain=100
  Saturation : Saturate  UpperLimit=100  LowerLimit=0
  HVBat_SOCcell043 : Outport
  wires:
  Voltage/1 -> Extended Kalman Filter - Multi Temperature EKF/2(Voltage)
  Current/1 -> Extended Kalman Filter - Multi Temperature EKF/1(Current)
  Temperature/1 -> Extended Kalman Filter - Multi Temperature EKF/3(Temperature)
  Extended Kalman Filter - Multi Temperature EKF/1(SOC) -> Gain/1
  Gain/1 -> Saturation/1
  Saturation/1 -> HVBat_SOCcell043/1, Data Store Write1/1

== system SOC_2023b/SOC/Multi-Temperature SOC EKF44 ==
  note: McMaster Automotive Research Centre - MARC
      | Electrical Engineer: Romulo Navega Vieira, M.Sc and MBA - PhD Candidate
  Current : Inport
  Voltage : Inport  Port=2
  Temperature : Inport  Port=3
  Data Store Write1 : DataStoreWrite  DataStoreName=HVBat_SOCcell044  IndexOptions=Index vector (dialog)  Indices=1  OutputSizes=1
  Extended Kalman Filter - Multi Temperature EKF : Subsystem
  Gain : Gain  Gain=100
  Saturation : Saturate  UpperLimit=100  LowerLimit=0
  HVBat_SOCcell044 : Outport
  wires:
  Voltage/1 -> Extended Kalman Filter - Multi Temperature EKF/2(Voltage)
  Current/1 -> Extended Kalman Filter - Multi Temperature EKF/1(Current)
  Temperature/1 -> Extended Kalman Filter - Multi Temperature EKF/3(Temperature)
  Extended Kalman Filter - Multi Temperature EKF/1(SOC) -> Gain/1
  Gain/1 -> Saturation/1
  Saturation/1 -> HVBat_SOCcell044/1, Data Store Write1/1

== system SOC_2023b/SOC/Multi-Temperature SOC EKF45 ==
  note: McMaster Automotive Research Centre - MARC
      | Electrical Engineer: Romulo Navega Vieira, M.Sc and MBA - PhD Candidate
  Current : Inport
  Voltage : Inport  Port=2
  Temperature : Inport  Port=3
  Data Store Write1 : DataStoreWrite  DataStoreName=HVBat_SOCcell045  IndexOptions=Index vector (dialog)  Indices=1  OutputSizes=1
  Extended Kalman Filter - Multi Temperature EKF : Subsystem
  Gain : Gain  Gain=100
  Saturation : Saturate  UpperLimit=100  LowerLimit=0
  HVBat_SOCcell045 : Outport
  wires:
  Voltage/1 -> Extended Kalman Filter - Multi Temperature EKF/2(Voltage)
  Current/1 -> Extended Kalman Filter - Multi Temperature EKF/1(Current)
  Temperature/1 -> Extended Kalman Filter - Multi Temperature EKF/3(Temperature)
  Extended Kalman Filter - Multi Temperature EKF/1(SOC) -> Gain/1
  Gain/1 -> Saturation/1
  Saturation/1 -> HVBat_SOCcell045/1, Data Store Write1/1

== system SOC_2023b/SOC/Multi-Temperature SOC EKF46 ==
  note: McMaster Automotive Research Centre - MARC
      | Electrical Engineer: Romulo Navega Vieira, M.Sc and MBA - PhD Candidate
  Current : Inport
  Voltage : Inport  Port=2
  Temperature : Inport  Port=3
  Data Store Write1 : DataStoreWrite  DataStoreName=HVBat_SOCcell046  IndexOptions=Index vector (dialog)  Indices=1  OutputSizes=1
  Extended Kalman Filter - Multi Temperature EKF : Subsystem
  Gain : Gain  Gain=100
  Saturation : Saturate  UpperLimit=100  LowerLimit=0
  HVBat_SOCcell046 : Outport
  wires:
  Voltage/1 -> Extended Kalman Filter - Multi Temperature EKF/2(Voltage)
  Current/1 -> Extended Kalman Filter - Multi Temperature EKF/1(Current)
  Temperature/1 -> Extended Kalman Filter - Multi Temperature EKF/3(Temperature)
  Extended Kalman Filter - Multi Temperature EKF/1(SOC) -> Gain/1
  Gain/1 -> Saturation/1
  Saturation/1 -> HVBat_SOCcell046/1, Data Store Write1/1

== system SOC_2023b/SOC/Multi-Temperature SOC EKF47 ==
  note: McMaster Automotive Research Centre - MARC
      | Electrical Engineer: Romulo Navega Vieira, M.Sc and MBA - PhD Candidate
  Current : Inport
  Voltage : Inport  Port=2
  Temperature : Inport  Port=3
  Data Store Write1 : DataStoreWrite  DataStoreName=HVBat_SOCcell047  IndexOptions=Index vector (dialog)  Indices=1  OutputSizes=1
  Extended Kalman Filter - Multi Temperature EKF : Subsystem
  Gain : Gain  Gain=100
  Saturation : Saturate  UpperLimit=100  LowerLimit=0
  HVBat_SOCcell047 : Outport
  wires:
  Voltage/1 -> Extended Kalman Filter - Multi Temperature EKF/2(Voltage)
  Current/1 -> Extended Kalman Filter - Multi Temperature EKF/1(Current)
  Temperature/1 -> Extended Kalman Filter - Multi Temperature EKF/3(Temperature)
  Extended Kalman Filter - Multi Temperature EKF/1(SOC) -> Gain/1
  Gain/1 -> Saturation/1
  Saturation/1 -> HVBat_SOCcell047/1, Data Store Write1/1

== system SOC_2023b/SOC/Multi-Temperature SOC EKF48 ==
  note: McMaster Automotive Research Centre - MARC
      | Electrical Engineer: Romulo Navega Vieira, M.Sc and MBA - PhD Candidate
  Current : Inport
  Voltage : Inport  Port=2
  Temperature : Inport  Port=3
  Data Store Write1 : DataStoreWrite  DataStoreName=HVBat_SOCcell048  IndexOptions=Index vector (dialog)  Indices=1  OutputSizes=1
  Extended Kalman Filter - Multi Temperature EKF : Subsystem
  Gain : Gain  Gain=100
  Saturation : Saturate  UpperLimit=100  LowerLimit=0
  HVBat_SOCcell048 : Outport
  wires:
  Voltage/1 -> Extended Kalman Filter - Multi Temperature EKF/2(Voltage)
  Current/1 -> Extended Kalman Filter - Multi Temperature EKF/1(Current)
  Temperature/1 -> Extended Kalman Filter - Multi Temperature EKF/3(Temperature)
  Extended Kalman Filter - Multi Temperature EKF/1(SOC) -> Gain/1
  Gain/1 -> Saturation/1
  Saturation/1 -> HVBat_SOCcell048/1, Data Store Write1/1

== system SOC_2023b/SOC/Multi-Temperature SOC EKF49 ==
  note: McMaster Automotive Research Centre - MARC
      | Electrical Engineer: Romulo Navega Vieira, M.Sc and MBA - PhD Candidate
  Current : Inport
  Voltage : Inport  Port=2
  Temperature : Inport  Port=3
  Data Store Write1 : DataStoreWrite  DataStoreName=HVBat_SOCcell049  IndexOptions=Index vector (dialog)  Indices=1  OutputSizes=1
  Extended Kalman Filter - Multi Temperature EKF : Subsystem
  Gain : Gain  Gain=100
  Saturation : Saturate  UpperLimit=100  LowerLimit=0
  HVBat_SOCcell049 : Outport
  wires:
  Voltage/1 -> Extended Kalman Filter - Multi Temperature EKF/2(Voltage)
  Current/1 -> Extended Kalman Filter - Multi Temperature EKF/1(Current)
  Temperature/1 -> Extended Kalman Filter - Multi Temperature EKF/3(Temperature)
  Extended Kalman Filter - Multi Temperature EKF/1(SOC) -> Gain/1
  Gain/1 -> Saturation/1
  Saturation/1 -> HVBat_SOCcell049/1, Data Store Write1/1

== system SOC_2023b/SOC/Multi-Temperature SOC EKF5 ==
  note: McMaster Automotive Research Centre - MARC
      | Electrical Engineer: Romulo Navega Vieira, M.Sc and MBA - PhD Candidate
  Current : Inport
  Voltage : Inport  Port=2
  Temperature : Inport  Port=3
  Data Store Write1 : DataStoreWrite  DataStoreName=HVBat_SOCcell005  IndexOptions=Index vector (dialog)  Indices=1  OutputSizes=1
  Extended Kalman Filter - Multi Temperature EKF : Subsystem
  Gain : Gain  Gain=100
  Saturation : Saturate  UpperLimit=100  LowerLimit=0
  HVBat_SOCcell005 : Outport
  wires:
  Voltage/1 -> Extended Kalman Filter - Multi Temperature EKF/2(Voltage)
  Current/1 -> Extended Kalman Filter - Multi Temperature EKF/1(Current)
  Temperature/1 -> Extended Kalman Filter - Multi Temperature EKF/3(Temperature)
  Extended Kalman Filter - Multi Temperature EKF/1(SOC) -> Gain/1
  Gain/1 -> Saturation/1
  Saturation/1 -> HVBat_SOCcell005/1, Data Store Write1/1

== system SOC_2023b/SOC/Multi-Temperature SOC EKF50 ==
  note: McMaster Automotive Research Centre - MARC
      | Electrical Engineer: Romulo Navega Vieira, M.Sc and MBA - PhD Candidate
  Current : Inport
  Voltage : Inport  Port=2
  Temperature : Inport  Port=3
  Data Store Write1 : DataStoreWrite  DataStoreName=HVBat_SOCcell050  IndexOptions=Index vector (dialog)  Indices=1  OutputSizes=1
  Extended Kalman Filter - Multi Temperature EKF : Subsystem
  Gain : Gain  Gain=100
  Saturation : Saturate  UpperLimit=100  LowerLimit=0
  HVBat_SOCcell050 : Outport
  wires:
  Voltage/1 -> Extended Kalman Filter - Multi Temperature EKF/2(Voltage)
  Current/1 -> Extended Kalman Filter - Multi Temperature EKF/1(Current)
  Temperature/1 -> Extended Kalman Filter - Multi Temperature EKF/3(Temperature)
  Extended Kalman Filter - Multi Temperature EKF/1(SOC) -> Gain/1
  Gain/1 -> Saturation/1
  Saturation/1 -> HVBat_SOCcell050/1, Data Store Write1/1
